simulink model "pfb_fft_vacc_float"  (R2016b)
settings: Solver=VariableStepAuto  StartTime=0.0  StopTime=1024*2^5  RelTol=1e-3
undefined symbols (not defined in the file): fft_stages
libraries: xbsIndex_r4 x15685, casper_library_misc x36, casper_library_delays x15, casper_library_sources x4, xbsReplacements_r4 x3, xps_library x1
(part 38 of 134)

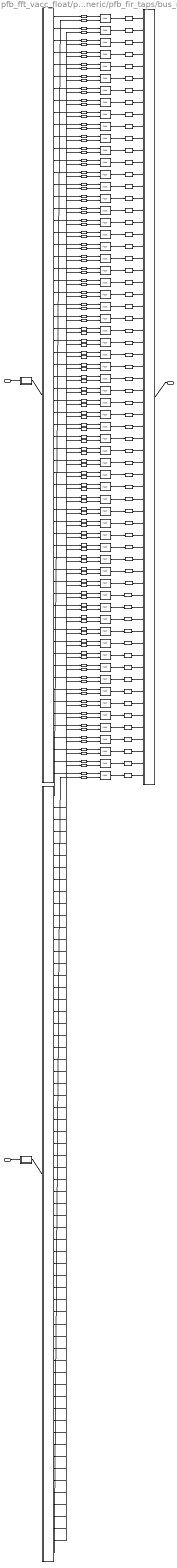
== system pfb_fft_vacc_float/pfb/pfb_fir_generic/pfb_fir_taps/bus_mult ==
  a : Inport
  b : Inport  Port=2
  Convert{,1..127} (128 alike) : xbsIndex_r4/Convert  gui_display_data_type=Floating-point  arith_type=Floating-point  n_bits=16  bin_pt=14  float_type=Single  exp_bits=8  fraction_bits=24  quantization=Truncate  overflow=Wrap  en=off  latency=4  pipeline=on  dbl_ovrd=off  block_type=convert
  Reinterpret{,1..63} (64 alike) : xbsIndex_r4/Reinterpret  force_arith_type=on  arith_type=Unsigned  force_bin_pt=on  bin_pt=0  block_type=reinterpret
  a*b_bussify : Subsystem  AncestorBlock=casper_library_flow_control/bus_create  mask(bus_create){inputNum=64}
      mask prompts: inputNum "Number of inputs:"
      mask init:
      | bus_create_init(gcb,...
      |     'inputNum', inputNum);
  a_debus : Subsystem  AncestorBlock=casper_library_flow_control/bus_expand  mask(bus_expand){mode=divisions of arbitrary size, outputNum=1, outputWidth=[10 10 10 10 10 10 10 10 10 10 10 10 10 10 10 10 10 10 10 10 10 10 10 10 10 10 10 10 10 10 10 10 10 10 10 10 10 10 10 10 10 10 10 10 10 10 10 10 10 10 10 10 10 10 10 10 10 10 10 10 10 10 10 10], outputBinaryPt=[9 9 9 9 9 9 9 9 9 9 9 9 9 9 9 9 9 9 9 9 9 9 9 9 9 9 9 9 9 9 9 9 9 9 9 9 9 9 9 9 9 9 9 9 9 9 9 9 9 9 9 9 9 9 9 9 9 9 9 9 9 9 9 9], outputArithmeticType=[1 1 1 1 1 1 1 1 1 1 1 1 1 1 1 1 1 1 1 1 1 1 1 1 1 1 1 1 1 1 1 1 1 1 1 1 1 1 1 1 1 1 1 1 1 1 1 1 1 1 1 1 1 1 1 1 1 1 1 1 1 1 1 1], show_format=on, outputToWorkspace=off, variablePrefix=, outputToModelAsWell=on}
      mask prompts: outputNum "Number of outputs:"; outputWidth "Output width [msb ... lsb]:"; outputBinaryPt "Output binary point position [msb ... lsb]:"; outputArithmeticType "Output arithmetic type (ufix=0, fix=1, bool=2) [msb ... lsb]:"; show_format "Print format string?"; outputToWorkspace "Output to workspace?"; variablePrefix "Variable name prefix:"; outputToModelAsWell "Output to model as well?"
      mask help:
      | Expands a 'bus' made using a bus_create or Xilinx
      | concat block.
      | The input will be divided into the specified number
      | of outputs with the specified arithmetic types.
      | Divisions are made from most significant bit.
      mask init: same as pfb_fft_vacc_float/bus_expand1
      settings: AttributesFormatString=64 outputs: f10.9,f10.9,f10.9,f10.9,f10.9,f10.9,f10.9,f10.9,f10.9,f10.9,f10.9,f10.9,f10.9,f10.9,f10.9,f10.9,f10.9,f10.9,f10.9,f10.9,f10.9,f10.9,f10.9,f10.9,f10.9,f10.9,f10.9,f10.9,f10.9,f10.9,f10.9,f10.9,f10.9,f10.9,f10.9,f10.9,f10.9,f10.9,f10.9,f10.9,f10.9,f10.9,f10.9,f10.9,f10.9,f10.9,f10.9,f10.9,f10.9,f10.9,f10.9,f10.9,f10.9,f10.9,f10.9,f10.9,f10.9,f10.9,f10.9,f10.9,f10.9,f10.9,f10.9,f10.9,
      mask TypeOptions mode: divisions of equal size | divisions of arbitrary size
      mask Callback mode: bus_expand_callback();
      mask Callback outputWidth: bus_expand_callback();
      mask Callback outputToWorkspace: bus_expand_callback();
  b_debus : Subsystem  AncestorBlock=casper_library_flow_control/bus_expand  mask(bus_expand){mode=divisions of arbitrary size, outputNum=1, outputWidth=[18 18 18 18 18 18 18 18 18 18 18 18 18 18 18 18 18 18 18 18 18 18 18 18 18 18 18 18 18 18 18 18 18 18 18 18 18 18 18 18 18 18 18 18 18 18 18 18 18 18 18 18 18 18 18 18 18 18 18 18 18 18 18 18], outputBinaryPt=[17 17 17 17 17 17 17 17 17 17 17 17 17 17 17 17 17 17 17 17 17 17 17 17 17 17 17 17 17 17 17 17 17 17 17 17 17 17 17 17 17 17 17 17 17 17 17 17 17 17 17 17 17 17 17 17 17 17 17 17 17 17 17 17], outputArithmeticType=[1 1 1 1 1 1 1 1 1 1 1 1 1 1 1 1 1 1 1 1 1 1 1 1 1 1 1 1 1 1 1 1 1 1 1 1 1 1 1 1 1 1 1 1 1 1 1 1 1 1 1 1 1 1 1 1 1 1 1 1 1 1 1 1], show_format=on, outputToWorkspace=off, variablePrefix=, outputToModelAsWell=on}
      mask prompts: outputNum "Number of outputs:"; outputWidth "Output width [msb ... lsb]:"; outputBinaryPt "Output binary point position [msb ... lsb]:"; outputArithmeticType "Output arithmetic type (ufix=0, fix=1, bool=2) [msb ... lsb]:"; show_format "Print format string?"; outputToWorkspace "Output to workspace?"; variablePrefix "Variable name prefix:"; outputToModelAsWell "Output to model as well?"
      mask help:
      | Expands a 'bus' made using a bus_create or Xilinx
      | concat block.
      | The input will be divided into the specified number
      | of outputs with the specified arithmetic types.
      | Divisions are made from most significant bit.
      mask init: same as pfb_fft_vacc_float/bus_expand1
      settings: AttributesFormatString=64 outputs: f18.17,f18.17,f18.17,f18.17,f18.17,f18.17,f18.17,f18.17,f18.17,f18.17,f18.17,f18.17,f18.17,f18.17,f18.17,f18.17,f18.17,f18.17,f18.17,f18.17,f18.17,f18.17,f18.17,f18.17,f18.17,f18.17,f18.17,f18.17,f18.17,f18.17,f18.17,f18.17,f18.17,f18.17,f18.17,f18.17,f18.17,f18.17,f18.17,f18.17,f18.17,f18.17,f18.17,f18.17,f18.17,f18.17,f18.17,f18.17,f18.17,f18.17,f18.17,f18.17,f18.17,f18.17,f18.17,f18.17,f18.17,f18.17,f18.17,f18.17,f18.17,f18.17,f18.17,f18.17,
      mask TypeOptions mode: divisions of equal size | divisions of arbitrary size
      mask Callback mode: bus_expand_callback();
      mask Callback outputWidth: bus_expand_callback();
      mask Callback outputToWorkspace: bus_expand_callback();
  mult{1..64} (64 alike) : xbsIndex_r4/Mult  precision=Full  arith_type="Signed  (2's comp)"  n_bits=28  bin_pt=26  quantization=Truncate  overflow=Wrap  en=off  latency=mult_latency+3  use_behavioral_HDL=off  opt=Speed  use_embedded=on  optimum_pipeline=off  pipeline=on  dbl_ovrd=off  use_rpm=on  placement_style=Triangular  block_type=mult
  repa : Subsystem  AncestorBlock=casper_library_bus/bus_replicate  mask(bus_replicate){replication=1, latency=0, misc=off, implementation=core}
      mask prompts: replication "replication factor"; misc "misc support"; implementation "delay implementation"
      mask help: Output bus formed by replicating input bus a number of times
      mask init:
      | bus_replicate_init(gcb, ...
      |     'replication', replication, ...
      |     'latency', latency, ...
      |     'implementation', implementation, ...
      |     'misc', misc);
      mask TypeOptions implementation: core | behavioral
  repb : Subsystem  AncestorBlock=casper_library_bus/bus_replicate  mask(bus_replicate){replication=1, latency=0, misc=off, implementation=core}
      mask prompts: replication "replication factor"; misc "misc support"; implementation "delay implementation"
      mask help: Output bus formed by replicating input bus a number of times
      mask init:
      | bus_replicate_init(gcb, ...
      |     'replication', replication, ...
      |     'latency', latency, ...
      |     'implementation', implementation, ...
      |     'misc', misc);
      mask TypeOptions implementation: core | behavioral
  a*b : Outport
  wires:
  a/1 -> repa/1(in)
  b/1 -> repb/1(in)
  repa/1(out) -> a_debus/1(bus_in)
  repb/1(out) -> b_debus/1(bus_in)
  a_debus/1(msb_out64) -> Convert/1
  b_debus/1(msb_out64) -> Convert1/1
  a_debus/2(out63) -> Convert2/1
  b_debus/2(out63) -> Convert3/1
  a_debus/3(out62) -> Convert4/1
  b_debus/3(out62) -> Convert5/1
  a_debus/4(out61) -> Convert6/1
  b_debus/4(out61) -> Convert7/1
  a_debus/5(out60) -> Convert8/1
  b_debus/5(out60) -> Convert9/1
  a_debus/6(out59) -> Convert10/1
  b_debus/6(out59) -> Convert11/1
  a_debus/7(out58) -> Convert12/1
  b_debus/7(out58) -> Convert13/1
  a_debus/8(out57) -> Convert14/1
  b_debus/8(out57) -> Convert15/1
  a_debus/9(out56) -> Convert16/1
  b_debus/9(out56) -> Convert17/1
  a_debus/10(out55) -> Convert24/1
  b_debus/10(out55) -> Convert25/1
  a_debus/11(out54) -> Convert26/1
  b_debus/11(out54) -> Convert27/1
  a_debus/12(out53) -> Convert28/1
  b_debus/12(out53) -> Convert29/1
  a_debus/13(out52) -> Convert30/1
  b_debus/13(out52) -> Convert31/1
  a_debus/14(out51) -> Convert18/1
  b_debus/14(out51) -> Convert19/1
  a_debus/15(out50) -> Convert20/1
  b_debus/15(out50) -> Convert21/1
  a_debus/16(out49) -> Convert22/1
  b_debus/16(out49) -> Convert23/1
  a_debus/17(out48) -> Convert32/1
  b_debus/17(out48) -> Convert33/1
  a_debus/18(out47) -> Convert40/1
  b_debus/18(out47) -> Convert41/1
  a_debus/19(out46) -> Convert42/1
  b_debus/19(out46) -> Convert43/1
  a_debus/20(out45) -> Convert44/1
  b_debus/20(out45) -> Convert45/1
  a_debus/21(out44) -> Convert46/1
  b_debus/21(out44) -> Convert47/1
  a_debus/22(out43) -> Convert34/1
  b_debus/22(out43) -> Convert35/1
  a_debus/23(out42) -> Convert36/1
  b_debus/23(out42) -> Convert37/1
  a_debus/24(out41) -> Convert38/1
  b_debus/24(out41) -> Convert39/1
  a_debus/25(out40) -> Convert48/1
  b_debus/25(out40) -> Convert49/1
  a_debus/26(out39) -> Convert56/1
  b_debus/26(out39) -> Convert57/1
  a_debus/27(out38) -> Convert58/1
  b_debus/27(out38) -> Convert59/1
  a_debus/28(out37) -> Convert60/1
  b_debus/28(out37) -> Convert61/1
  a_debus/29(out36) -> Convert62/1
  b_debus/29(out36) -> Convert63/1
  a_debus/30(out35) -> Convert50/1
  b_debus/30(out35) -> Convert51/1
  a_debus/31(out34) -> Convert52/1
  b_debus/31(out34) -> Convert53/1
  a_debus/32(out33) -> Convert54/1
  b_debus/32(out33) -> Convert55/1
  a_debus/33(out32) -> Convert64/1
  b_debus/33(out32) -> Convert65/1
  a_debus/34(out31) -> Convert72/1
  b_debus/34(out31) -> Convert73/1
  a_debus/35(out30) -> Convert74/1
  b_debus/35(out30) -> Convert75/1
  a_debus/36(out29) -> Convert76/1
  b_debus/36(out29) -> Convert77/1
  a_debus/37(out28) -> Convert78/1
  b_debus/37(out28) -> Convert79/1
  a_debus/38(out27) -> Convert66/1
  b_debus/38(out27) -> Convert67/1
  a_debus/39(out26) -> Convert68/1
  b_debus/39(out26) -> Convert69/1
  a_debus/40(out25) -> Convert70/1
  b_debus/40(out25) -> Convert71/1
  a_debus/41(out24) -> Convert80/1
  b_debus/41(out24) -> Convert81/1
  a_debus/42(out23) -> Convert88/1
  b_debus/42(out23) -> Convert89/1
  a_debus/43(out22) -> Convert90/1
  b_debus/43(out22) -> Convert91/1
  a_debus/44(out21) -> Convert92/1
  b_debus/44(out21) -> Convert93/1
  a_debus/45(out20) -> Convert94/1
  b_debus/45(out20) -> Convert95/1
  a_debus/46(out19) -> Convert82/1
  b_debus/46(out19) -> Convert83/1
  a_debus/47(out18) -> Convert84/1
  b_debus/47(out18) -> Convert85/1
  a_debus/48(out17) -> Convert86/1
  b_debus/48(out17) -> Convert87/1
  a_debus/49(out16) -> Convert96/1
  b_debus/49(out16) -> Convert97/1
  a_debus/50(out15) -> Convert104/1
  b_debus/50(out15) -> Convert105/1
  a_debus/51(out14) -> Convert106/1
  b_debus/51(out14) -> Convert107/1
  a_debus/52(out13) -> Convert108/1
  b_debus/52(out13) -> Convert109/1
  a_debus/53(out12) -> Convert110/1
  b_debus/53(out12) -> Convert111/1
  a_debus/54(out11) -> Convert98/1
  b_debus/54(out11) -> Convert99/1
  a_debus/55(out10) -> Convert100/1
  b_debus/55(out10) -> Convert101/1
  a_debus/56(out9) -> Convert102/1
  b_debus/56(out9) -> Convert103/1
  a_debus/57(out8) -> Convert124/1
  b_debus/57(out8) -> Convert125/1
  a_debus/58(out7) -> Convert116/1
  b_debus/58(out7) -> Convert117/1
  a_debus/59(out6) -> Convert118/1
  b_debus/59(out6) -> Convert119/1
  a_debus/60(out5) -> Convert120/1
  b_debus/60(out5) -> Convert121/1
  a_debus/61(out4) -> Convert122/1
  b_debus/61(out4) -> Convert123/1
  a_debus/62(out3) -> Convert126/1
  b_debus/62(out3) -> Convert127/1
  a_debus/63(out2) -> Convert112/1
  b_debus/63(out2) -> Convert113/1
  a_debus/64(lsb_out1) -> Convert114/1
  b_debus/64(lsb_out1) -> Convert115/1
  mult2/1 -> Reinterpret1/1
  mult3/1 -> Reinterpret2/1
  mult4/1 -> Reinterpret3/1
  mult5/1 -> Reinterpret4/1
  mult6/1 -> Reinterpret5/1
  mult7/1 -> Reinterpret6/1
  mult8/1 -> Reinterpret7/1
  mult9/1 -> Reinterpret8/1
  mult10/1 -> Reinterpret9/1
  mult11/1 -> Reinterpret10/1
  mult12/1 -> Reinterpret11/1
  mult13/1 -> Reinterpret12/1
  mult14/1 -> Reinterpret13/1
  mult15/1 -> Reinterpret14/1
  mult16/1 -> Reinterpret15/1
  mult24/1 -> Reinterpret22/1
  mult17/1 -> Reinterpret23/1
  mult18/1 -> Reinterpret16/1
  mult19/1 -> Reinterpret17/1
  mult20/1 -> Reinterpret18/1
  mult21/1 -> Reinterpret19/1
  mult22/1 -> Reinterpret20/1
  mult23/1 -> Reinterpret21/1
  mult32/1 -> Reinterpret30/1
  mult25/1 -> Reinterpret31/1
  mult26/1 -> Reinterpret24/1
  mult27/1 -> Reinterpret25/1
  mult28/1 -> Reinterpret26/1
  mult29/1 -> Reinterpret27/1
  mult30/1 -> Reinterpret28/1
  mult31/1 -> Reinterpret29/1
  mult40/1 -> Reinterpret38/1
  mult33/1 -> Reinterpret39/1
  mult34/1 -> Reinterpret32/1
  mult35/1 -> Reinterpret33/1
  mult36/1 -> Reinterpret34/1
  mult37/1 -> Reinterpret35/1
  mult38/1 -> Reinterpret36/1
  mult39/1 -> Reinterpret37/1
  mult48/1 -> Reinterpret46/1
  mult41/1 -> Reinterpret47/1
  mult42/1 -> Reinterpret40/1
  mult43/1 -> Reinterpret41/1
  mult44/1 -> Reinterpret42/1
  mult45/1 -> Reinterpret43/1
  mult46/1 -> Reinterpret44/1
  mult47/1 -> Reinterpret45/1
  mult56/1 -> Reinterpret54/1
  mult49/1 -> Reinterpret55/1
  mult50/1 -> Reinterpret48/1
  mult51/1 -> Reinterpret49/1
  mult52/1 -> Reinterpret50/1
  mult53/1 -> Reinterpret51/1
  mult54/1 -> Reinterpret52/1
  mult55/1 -> Reinterpret53/1
  mult57/1 -> Reinterpret62/1
  mult58/1 -> Reinterpret63/1
  mult59/1 -> Reinterpret56/1
  mult60/1 -> Reinterpret57/1
  mult61/1 -> Reinterpret58/1
  mult62/1 -> Reinterpret59/1
  mult63/1 -> Reinterpret60/1
  mult64/1 -> Reinterpret61/1
  a*b_bussify/1(bus_out) -> a*b/1
  Convert/1 -> mult1/1
  Convert1/1 -> mult1/2
  Convert3/1 -> mult2/2
  Convert2/1 -> mult2/1
  Convert5/1 -> mult3/2
  Convert4/1 -> mult3/1
  Convert7/1 -> mult4/2
  Convert6/1 -> mult4/1
  Convert14/1 -> mult8/1
  Convert11/1 -> mult6/2
  Convert10/1 -> mult6/1
  Convert13/1 -> mult7/2
  Convert12/1 -> mult7/1
  Convert8/1 -> mult5/1
  Convert15/1 -> mult8/2
  Convert9/1 -> mult5/2
  Convert31/1 -> mult13/2
  Convert19/1 -> mult14/2
  Convert16/1 -> mult9/1
  Convert17/1 -> mult9/2
  Convert26/1 -> mult11/1
  Convert29/1 -> mult12/2
  Convert18/1 -> mult14/1
  Convert23/1 -> mult16/2
  Convert22/1 -> mult16/1
  Convert27/1 -> mult11/2
  Convert21/1 -> mult15/2
  Convert24/1 -> mult10/1
  Convert20/1 -> mult15/1
  Convert28/1 -> mult12/1
  Convert25/1 -> mult10/2
  Convert30/1 -> mult13/1
  Convert33/1 -> mult24/2
  Convert45/1 -> mult19/2
  Convert38/1 -> mult23/1
  Convert41/1 -> mult17/2
  Convert34/1 -> mult21/1
  Convert46/1 -> mult20/1
  Convert32/1 -> mult24/1
  Convert43/1 -> mult18/2
  Convert39/1 -> mult23/2
  Convert37/1 -> mult22/2
  Convert42/1 -> mult18/1
  Convert40/1 -> mult17/1
  Convert36/1 -> mult22/1
  Convert44/1 -> mult19/1
  Convert47/1 -> mult20/2
  Convert35/1 -> mult21/2
  Convert55/1 -> mult31/2
  Convert61/1 -> mult27/2
  Convert58/1 -> mult26/1
  Convert52/1 -> mult30/1
  Convert48/1 -> mult32/1
  Convert51/1 -> mult29/2
  Convert53/1 -> mult30/2
  Convert62/1 -> mult28/1
  Convert49/1 -> mult32/2
  Convert60/1 -> mult27/1
  Convert56/1 -> mult25/1
  Convert57/1 -> mult25/2
  Convert54/1 -> mult31/1
  Convert59/1 -> mult26/2
  Convert50/1 -> mult29/1
  Convert63/1 -> mult28/2
  Convert79/1 -> mult36/2
  Convert74/1 -> mult34/1
  Convert78/1 -> mult36/1
  Convert77/1 -> mult35/2
  Convert65/1 -> mult40/2
  Convert71/1 -> mult39/2
  Convert64/1 -> mult40/1
  Convert69/1 -> mult38/2
  Convert70/1 -> mult39/1
  Convert76/1 -> mult35/1
  Convert66/1 -> mult37/1
  Convert67/1 -> mult37/2
  Convert68/1 -> mult38/1
  Convert73/1 -> mult33/2
  Convert75/1 -> mult34/2
  Convert72/1 -> mult33/1
  Convert80/1 -> mult48/1
  Convert91/1 -> mult42/2
  Convert89/1 -> mult41/2
  Convert87/1 -> mult47/2
  Convert94/1 -> mult44/1
  Convert88/1 -> mult41/1
  Convert93/1 -> mult43/2
  Convert95/1 -> mult44/2
  Convert81/1 -> mult48/2
  Convert92/1 -> mult43/1
  Convert86/1 -> mult47/1
  Convert84/1 -> mult46/1
  Convert85/1 -> mult46/2
  Convert90/1 -> mult42/1
  Convert83/1 -> mult45/2
  Convert82/1 -> mult45/1
  Convert108/1 -> mult51/1
  Convert106/1 -> mult50/1
  Convert98/1 -> mult53/1
  Convert102/1 -> mult55/1
  Convert104/1 -> mult49/1
  Convert105/1 -> mult49/2
  Convert110/1 -> mult52/1
  Convert109/1 -> mult51/2
  Convert111/1 -> mult52/2
  Convert100/1 -> mult54/1
  Convert96/1 -> mult56/1
  Convert97/1 -> mult56/2
  Convert107/1 -> mult50/2
  Convert101/1 -> mult54/2
  Convert103/1 -> mult55/2
  Convert99/1 -> mult53/2
  Convert126/1 -> mult62/1
  Convert127/1 -> mult62/2
  Convert115/1 -> mult64/2
  Convert118/1 -> mult59/1
  Convert116/1 -> mult58/1
  Convert117/1 -> mult58/2
  Convert125/1 -> mult57/2
  Convert114/1 -> mult64/1
  Convert119/1 -> mult59/2
  Convert122/1 -> mult61/1
  Convert112/1 -> mult63/1
  Convert121/1 -> mult60/2
  Convert124/1 -> mult57/1
  Convert123/1 -> mult61/2
  Convert120/1 -> mult60/1
  Convert113/1 -> mult63/2
  Reinterpret/1 -> a*b_bussify/1(in1)
  Reinterpret1/1 -> a*b_bussify/2(in2)
  Reinterpret2/1 -> a*b_bussify/3(in3)
  Reinterpret3/1 -> a*b_bussify/4(in4)
  Reinterpret4/1 -> a*b_bussify/5(in5)
  Reinterpret7/1 -> a*b_bussify/8(in8)
  Reinterpret6/1 -> a*b_bussify/7(in7)
  Reinterpret5/1 -> a*b_bussify/6(in6)
  Reinterpret13/1 -> a*b_bussify/14(in14)
  Reinterpret15/1 -> a*b_bussify/16(in16)
  Reinterpret11/1 -> a*b_bussify/12(in12)
  Reinterpret9/1 -> a*b_bussify/10(in10)
  Reinterpret14/1 -> a*b_bussify/15(in15)
  Reinterpret8/1 -> a*b_bussify/9(in9)
  Reinterpret12/1 -> a*b_bussify/13(in13)
  Reinterpret10/1 -> a*b_bussify/11(in11)
  Reinterpret16/1 -> a*b_bussify/19(in19)
  Reinterpret21/1 -> a*b_bussify/24(in24)
  Reinterpret17/1 -> a*b_bussify/20(in20)
  Reinterpret18/1 -> a*b_bussify/21(in21)
  Reinterpret22/1 -> a*b_bussify/17(in17)
  Reinterpret23/1 -> a*b_bussify/18(in18)
  Reinterpret20/1 -> a*b_bussify/23(in23)
  Reinterpret19/1 -> a*b_bussify/22(in22)
  Reinterpret24/1 -> a*b_bussify/27(in27)
  Reinterpret30/1 -> a*b_bussify/25(in25)
  Reinterpret26/1 -> a*b_bussify/29(in29)
  Reinterpret27/1 -> a*b_bussify/30(in30)
  Reinterpret28/1 -> a*b_bussify/31(in31)
  Reinterpret31/1 -> a*b_bussify/26(in26)
  Reinterpret29/1 -> a*b_bussify/32(in32)
  Reinterpret25/1 -> a*b_bussify/28(in28)
  Reinterpret34/1 -> a*b_bussify/37(in37)
  Reinterpret36/1 -> a*b_bussify/39(in39)
  Reinterpret35/1 -> a*b_bussify/38(in38)
  Reinterpret38/1 -> a*b_bussify/33(in33)
  Reinterpret32/1 -> a*b_bussify/35(in35)
  Reinterpret33/1 -> a*b_bussify/36(in36)
  Reinterpret37/1 -> a*b_bussify/40(in40)
  Reinterpret39/1 -> a*b_bussify/34(in34)
  Reinterpret41/1 -> a*b_bussify/44(in44)
  Reinterpret44/1 -> a*b_bussify/47(in47)
  Reinterpret42/1 -> a*b_bussify/45(in45)
  Reinterpret46/1 -> a*b_bussify/41(in41)
  Reinterpret40/1 -> a*b_bussify/43(in43)
  Reinterpret47/1 -> a*b_bussify/42(in42)
  Reinterpret45/1 -> a*b_bussify/48(in48)
  Reinterpret43/1 -> a*b_bussify/46(in46)
  Reinterpret51/1 -> a*b_bussify/54(in54)
  Reinterpret54/1 -> a*b_bussify/49(in49)
  Reinterpret55/1 -> a*b_bussify/50(in50)
  Reinterpret48/1 -> a*b_bussify/51(in51)
  Reinterpret53/1 -> a*b_bussify/56(in56)
  Reinterpret50/1 -> a*b_bussify/53(in53)
  Reinterpret52/1 -> a*b_bussify/55(in55)
  Reinterpret49/1 -> a*b_bussify/52(in52)
  Reinterpret63/1 -> a*b_bussify/58(in58)
  Reinterpret56/1 -> a*b_bussify/59(in59)
  Reinterpret61/1 -> a*b_bussify/64(in64)
  Reinterpret60/1 -> a*b_bussify/63(in63)
  Reinterpret57/1 -> a*b_bussify/60(in60)
  Reinterpret59/1 -> a*b_bussify/62(in62)
  Reinterpret62/1 -> a*b_bussify/57(in57)
  Reinterpret58/1 -> a*b_bussify/61(in61)
  mult1/1 -> Reinterpret/1

== system pfb_fft_vacc_float/pfb/pfb_fir_generic/pfb_fir_taps/pipeline1 ==
  d : Inport
  Register0 : xbsIndex_r4/Register  init=0  rst=off  en=off  dbl_ovrd=off  block_type=register
      settings: block_version=11.4
  Register1 : xbsIndex_r4/Register  init=0  rst=off  en=off  dbl_ovrd=off  block_type=register
  q : Outport
  wires:
  Register1/1 -> q/1
  d/1 -> Register0/1
  Register0/1 -> Register1/1

== system pfb_fft_vacc_float/pfb/pfb_fir_generic/pfb_fir_taps/pipeline2 ==
  d : Inport
  Register0 : xbsIndex_r4/Register  init=0  rst=off  en=off  dbl_ovrd=off  block_type=register
      settings: block_version=11.4
  Register1 : xbsIndex_r4/Register  init=0  rst=off  en=off  dbl_ovrd=off  block_type=register
  q : Outport
  wires:
  Register0/1 -> Register1/1
  d/1 -> Register0/1
  Register1/1 -> q/1

== system pfb_fft_vacc_float/pfb/pfb_fir_generic/pfb_fir_taps/pipeline3 ==
  d : Inport
  Register0 : xbsIndex_r4/Register  init=0  rst=off  en=off  dbl_ovrd=off  block_type=register
      settings: block_version=11.4
  Register1 : xbsIndex_r4/Register  init=0  rst=off  en=off  dbl_ovrd=off  block_type=register
  q : Outport
  wires:
  Register1/1 -> q/1
  d/1 -> Register0/1
  Register0/1 -> Register1/1

== system pfb_fft_vacc_float/pfb/pfb_fir_generic/pfb_fir_taps/pipeline8 ==
  d : Inport
  Register0 : xbsIndex_r4/Register  init=0  rst=off  en=off  dbl_ovrd=off  block_type=register
      settings: block_version=11.4
  Register1 : xbsIndex_r4/Register  init=0  rst=off  en=off  dbl_ovrd=off  block_type=register
  q : Outport
  wires:
  Register0/1 -> Register1/1
  d/1 -> Register0/1
  Register1/1 -> q/1

== system pfb_fft_vacc_float/pfb/pfb_fir_generic/pfb_fir_taps/tap_delays ==
  addra : Inport
  dina : Inport  Port=2
  wea : Inport  Port=3
  addrb : Inport  Port=4
  dinb : Inport  Port=5
  web : Inport  Port=6
  A_bussify0 : Subsystem  AncestorBlock=casper_library_flow_control/bus_create  mask(bus_create){inputNum=14}
      mask prompts: inputNum "Number of inputs:"
      mask init:
      | bus_create_init(gcb,...
      |     'inputNum', inputNum);
  B_bussify0 : Subsystem  AncestorBlock=casper_library_flow_control/bus_create  mask(bus_create){inputNum=14}
      mask prompts: inputNum "Number of inputs:"
      mask init:
      | bus_create_init(gcb,...
      |     'inputNum', inputNum);
  alsbs_slice : xbsIndex_r4/Slice  nbits=10  boolean_output=off  mode=Lower Bit Location + Width  bit1=0  base1=LSB of Input  bit0=0  base0=LSB of Input  dbl_ovrd=off  block_type=slice
  blsbs_slice : xbsIndex_r4/Slice  nbits=10  boolean_output=off  mode=Lower Bit Location + Width  bit1=0  base1=LSB of Input  bit0=0  base0=LSB of Input  dbl_ovrd=off  block_type=slice
  bram0_{0..13} (14 alike) : xbsIndex_r4/Dual Port RAM  depth=1024  initVector=getfield(get_param(gcb, 'UserData'), 'initVector')  distributed_mem=Block RAM  init_a=0  init_b=0  rst_a=off  rst_b=off  en_a=off  en_b=off  latency=4  write_mode_A=Read Before Write  write_mode_B=Read Before Write  optimize=Speed  dbl_ovrd=off  block_type=dpram
  ddina0 : xbsIndex_r4/Delay  rst=off  infoeditControl=Selection of Reset will increase slice count due to use of real FFs and instead of SRLs  en=off  latency=0  reg_retiming=on  dbl_ovrd=off  block_type=delay
      settings: infoedit=Hardware notes: A delay line is a chain, each link of which is an SRL16 or SRL32 followed by a flip-flop.
  ddinb0 : xbsIndex_r4/Delay  rst=off  infoeditControl=Selection of Reset will increase slice count due to use of real FFs and instead of SRLs  en=off  latency=6  reg_retiming=off  dbl_ovrd=off  block_type=delay
      settings: infoedit=Hardware notes: A delay line is a chain, each link of which is an SRL16 or SRL32 followed by a flip-flop.
  debus_addra0 : Subsystem  AncestorBlock=casper_library_flow_control/bus_expand  mask(bus_expand){mode=divisions of equal size, outputNum=14, outputWidth=[10 10 10 10 10 10 10 10 10 10 10 10 10 10], outputBinaryPt=[0 0 0 0 0 0 0 0 0 0 0 0 0 0], outputArithmeticType=[0 0 0 0 0 0 0 0 0 0 0 0 0 0], show_format=on, outputToWorkspace=off, variablePrefix=, outputToModelAsWell=on}
      mask prompts: outputNum "Number of outputs:"; outputWidth "Output width:"; outputBinaryPt "Output binary point position:"; outputArithmeticType "Output arithmetic type (ufix=0, fix=1, bool=2):"; show_format "Print format string?"; outputToWorkspace "Output to workspace?"; variablePrefix "Variable name prefix:"; outputToModelAsWell "Output to model as well?"
      mask help:
      | Expands a 'bus' made using a bus_create or Xilinx
      | concat block.
      | The input will be divided into the specified number
      | of outputs with the specified arithmetic types.
      | Divisions are made from most significant bit.
      mask init: same as pfb_fft_vacc_float/bus_expand1
      settings: AttributesFormatString=14 outputs: uf10.0,uf10.0,uf10.0,uf10.0,uf10.0,uf10.0,uf10.0,uf10.0,uf10.0,uf10.0,uf10.0,uf10.0,uf10.0,uf10.0,
      mask TypeOptions mode: divisions of equal size | divisions of arbitrary size
      mask Callback mode: bus_expand_callback();
      mask Callback outputWidth: bus_expand_callback();
      mask Callback outputToWorkspace: bus_expand_callback();
  debus_addrb0 : Subsystem  AncestorBlock=casper_library_flow_control/bus_expand  mask(bus_expand){mode=divisions of equal size, outputNum=14, outputWidth=[10 10 10 10 10 10 10 10 10 10 10 10 10 10], outputBinaryPt=[0 0 0 0 0 0 0 0 0 0 0 0 0 0], outputArithmeticType=[0 0 0 0 0 0 0 0 0 0 0 0 0 0], show_format=on, outputToWorkspace=off, variablePrefix=, outputToModelAsWell=on}
      mask prompts: outputNum "Number of outputs:"; outputWidth "Output width:"; outputBinaryPt "Output binary point position:"; outputArithmeticType "Output arithmetic type (ufix=0, fix=1, bool=2):"; show_format "Print format string?"; outputToWorkspace "Output to workspace?"; variablePrefix "Variable name prefix:"; outputToModelAsWell "Output to model as well?"
      mask help:
      | Expands a 'bus' made using a bus_create or Xilinx
      | concat block.
      | The input will be divided into the specified number
      | of outputs with the specified arithmetic types.
      | Divisions are made from most significant bit.
      mask init: same as pfb_fft_vacc_float/bus_expand1
      settings: AttributesFormatString=14 outputs: uf10.0,uf10.0,uf10.0,uf10.0,uf10.0,uf10.0,uf10.0,uf10.0,uf10.0,uf10.0,uf10.0,uf10.0,uf10.0,uf10.0,
      mask TypeOptions mode: divisions of equal size | divisions of arbitrary size
      mask Callback mode: bus_expand_callback();
      mask Callback outputWidth: bus_expand_callback();
      mask Callback outputToWorkspace: bus_expand_callback();
  debus_dina0 : Subsystem  AncestorBlock=casper_library_flow_control/bus_expand  mask(bus_expand){mode=divisions of arbitrary size, outputNum=1, outputWidth=[12 36 36 36 36 36 36 36 36 36 36 36 36 36], outputBinaryPt=[0 0 0 0 0 0 0 0 0 0 0 0 0 0], outputArithmeticType=[0 0 0 0 0 0 0 0 0 0 0 0 0 0], show_format=on, outputToWorkspace=off, variablePrefix=, outputToModelAsWell=on}
      mask prompts: outputNum "Number of outputs:"; outputWidth "Output width [msb ... lsb]:"; outputBinaryPt "Output binary point position [msb ... lsb]:"; outputArithmeticType "Output arithmetic type (ufix=0, fix=1, bool=2) [msb ... lsb]:"; show_format "Print format string?"; outputToWorkspace "Output to workspace?"; variablePrefix "Variable name prefix:"; outputToModelAsWell "Output to model as well?"
      mask help:
      | Expands a 'bus' made using a bus_create or Xilinx
      | concat block.
      | The input will be divided into the specified number
      | of outputs with the specified arithmetic types.
      | Divisions are made from most significant bit.
      mask init: same as pfb_fft_vacc_float/bus_expand1
      settings: AttributesFormatString=14 outputs: uf12.0,uf36.0,uf36.0,uf36.0,uf36.0,uf36.0,uf36.0,uf36.0,uf36.0,uf36.0,uf36.0,uf36.0,uf36.0,uf36.0,
      mask TypeOptions mode: divisions of equal size | divisions of arbitrary size
      mask Callback mode: bus_expand_callback();
      mask Callback outputWidth: bus_expand_callback();
      mask Callback outputToWorkspace: bus_expand_callback();
  debus_dinb0 : Subsystem  AncestorBlock=casper_library_flow_control/bus_expand  mask(bus_expand){mode=divisions of arbitrary size, outputNum=1, outputWidth=[12 36 36 36 36 36 36 36 36 36 36 36 36 36], outputBinaryPt=[0 0 0 0 0 0 0 0 0 0 0 0 0 0], outputArithmeticType=[0 0 0 0 0 0 0 0 0 0 0 0 0 0], show_format=on, outputToWorkspace=off, variablePrefix=, outputToModelAsWell=on}
      mask prompts: outputNum "Number of outputs:"; outputWidth "Output width [msb ... lsb]:"; outputBinaryPt "Output binary point position [msb ... lsb]:"; outputArithmeticType "Output arithmetic type (ufix=0, fix=1, bool=2) [msb ... lsb]:"; show_format "Print format string?"; outputToWorkspace "Output to workspace?"; variablePrefix "Variable name prefix:"; outputToModelAsWell "Output to model as well?"
      mask help:
      | Expands a 'bus' made using a bus_create or Xilinx
      | concat block.
      | The input will be divided into the specified number
      | of outputs with the specified arithmetic types.
      | Divisions are made from most significant bit.
      mask init: same as pfb_fft_vacc_float/bus_expand1
      settings: AttributesFormatString=14 outputs: uf12.0,uf36.0,uf36.0,uf36.0,uf36.0,uf36.0,uf36.0,uf36.0,uf36.0,uf36.0,uf36.0,uf36.0,uf36.0,uf36.0,
      mask TypeOptions mode: divisions of equal size | divisions of arbitrary size
      mask Callback mode: bus_expand_callback();
      mask Callback outputWidth: bus_expand_callback();
      mask Callback outputToWorkspace: bus_expand_callback();
  debus_wea0 : Subsystem  AncestorBlock=casper_library_flow_control/bus_expand  mask(bus_expand){mode=divisions of equal size, outputNum=14, outputWidth=[1 1 1 1 1 1 1 1 1 1 1 1 1 1], outputBinaryPt=[0 0 0 0 0 0 0 0 0 0 0 0 0 0], outputArithmeticType=[2 2 2 2 2 2 2 2 2 2 2 2 2 2], show_format=on, outputToWorkspace=off, variablePrefix=, outputToModelAsWell=on}
      mask prompts: outputNum "Number of outputs:"; outputWidth "Output width:"; outputBinaryPt "Output binary point position:"; outputArithmeticType "Output arithmetic type (ufix=0, fix=1, bool=2):"; show_format "Print format string?"; outputToWorkspace "Output to workspace?"; variablePrefix "Variable name prefix:"; outputToModelAsWell "Output to model as well?"
      mask help:
      | Expands a 'bus' made using a bus_create or Xilinx
      | concat block.
      | The input will be divided into the specified number
      | of outputs with the specified arithmetic types.
      | Divisions are made from most significant bit.
      mask init: same as pfb_fft_vacc_float/bus_expand1
      settings: AttributesFormatString=14 outputs: b,b,b,b,b,b,b,b,b,b,b,b,b,b,
      mask TypeOptions mode: divisions of equal size | divisions of arbitrary size
      mask Callback mode: bus_expand_callback();
      mask Callback outputWidth: bus_expand_callback();
      mask Callback outputToWorkspace: bus_expand_callback();
  debus_web0 : Subsystem  AncestorBlock=casper_library_flow_control/bus_expand  mask(bus_expand){mode=divisions of equal size, outputNum=14, outputWidth=[1 1 1 1 1 1 1 1 1 1 1 1 1 1], outputBinaryPt=[0 0 0 0 0 0 0 0 0 0 0 0 0 0], outputArithmeticType=[2 2 2 2 2 2 2 2 2 2 2 2 2 2], show_format=on, outputToWorkspace=off, variablePrefix=, outputToModelAsWell=on}
      mask prompts: outputNum "Number of outputs:"; outputWidth "Output width:"; outputBinaryPt "Output binary point position:"; outputArithmeticType "Output arithmetic type (ufix=0, fix=1, bool=2):"; show_format "Print format string?"; outputToWorkspace "Output to workspace?"; variablePrefix "Variable name prefix:"; outputToModelAsWell "Output to model as well?"
      mask help:
      | Expands a 'bus' made using a bus_create or Xilinx
      | concat block.
      | The input will be divided into the specified number
      | of outputs with the specified arithmetic types.
      | Divisions are made from most significant bit.
      mask init: same as pfb_fft_vacc_float/bus_expand1
      settings: AttributesFormatString=14 outputs: b,b,b,b,b,b,b,b,b,b,b,b,b,b,
      mask TypeOptions mode: divisions of equal size | divisions of arbitrary size
      mask Callback mode: bus_expand_callback();
      mask Callback outputWidth: bus_expand_callback();
      mask Callback outputToWorkspace: bus_expand_callback();
  rep_addra0 : Subsystem  AncestorBlock=casper_library_bus/bus_replicate  mask(bus_replicate){replication=14, latency=6, misc=off, implementation=core}
      mask prompts: replication "replication factor"; misc "misc support"; implementation "delay implementation"
      mask help: Output bus formed by replicating input bus a number of times
      mask init:
      | bus_replicate_init(gcb, ...
      |     'replication', replication, ...
      |     'latency', latency, ...
      |     'implementation', implementation, ...
      |     'misc', misc);
      mask TypeOptions implementation: core | behavioral
  rep_addrb0 : Subsystem  AncestorBlock=casper_library_bus/bus_replicate  mask(bus_replicate){replication=14, latency=6, misc=off, implementation=core}
      mask prompts: replication "replication factor"; misc "misc support"; implementation "delay implementation"
      mask help: Output bus formed by replicating input bus a number of times
      mask init:
      | bus_replicate_init(gcb, ...
      |     'replication', replication, ...
      |     'latency', latency, ...
      |     'implementation', implementation, ...
      |     'misc', misc);
      mask TypeOptions implementation: core | behavioral
  rep_wea0 : Subsystem  AncestorBlock=casper_library_bus/bus_replicate  mask(bus_replicate){replication=14, latency=0, misc=off, implementation=behavioral}
      mask prompts: replication "replication factor"; misc "misc support"; implementation "delay implementation"
      mask help: Output bus formed by replicating input bus a number of times
      mask init:
      | bus_replicate_init(gcb, ...
      |     'replication', replication, ...
      |     'latency', latency, ...
      |     'implementation', implementation, ...
      |     'misc', misc);
      mask TypeOptions implementation: core | behavioral
  rep_web0 : Subsystem  AncestorBlock=casper_library_bus/bus_replicate  mask(bus_replicate){replication=14, latency=6, misc=off, implementation=core}
      mask prompts: replication "replication factor"; misc "misc support"; implementation "delay implementation"
      mask help: Output bus formed by replicating input bus a number of times
      mask init:
      | bus_replicate_init(gcb, ...
      |     'replication', replication, ...
      |     'latency', latency, ...
      |     'implementation', implementation, ...
      |     'misc', misc);
      mask TypeOptions implementation: core | behavioral
  A : Outport
  B : Outport  Port=2
  wires:
  addrb/1 -> blsbs_slice/1
  addra/1 -> alsbs_slice/1
  blsbs_slice/1 -> rep_addrb0/1(in)
  alsbs_slice/1 -> rep_addra0/1(in)
  dina/1 -> ddina0/1
  wea/1 -> rep_wea0/1(in)
  dinb/1 -> ddinb0/1
  web/1 -> rep_web0/1(in)
  rep_addrb0/1(out) -> debus_addrb0/1(bus_in)
  rep_addra0/1(out) -> debus_addra0/1(bus_in)
  ddina0/1 -> debus_dina0/1(bus_in)
  rep_wea0/1(out) -> debus_wea0/1(bus_in)
  ddinb0/1 -> debus_dinb0/1(bus_in)
  rep_web0/1(out) -> debus_web0/1(bus_in)
  debus_addra0/1(msb_out14) -> bram0_0/1
  debus_dina0/1(msb_out14) -> bram0_0/2
  debus_wea0/1(msb_out14) -> bram0_0/3
  debus_addrb0/1(msb_out14) -> bram0_0/4
  debus_dinb0/1(msb_out14) -> bram0_0/5
  debus_web0/1(msb_out14) -> bram0_0/6
  debus_addra0/2(out13) -> bram0_1/1
  debus_dina0/2(out13) -> bram0_1/2
  debus_wea0/2(out13) -> bram0_1/3
  debus_addrb0/2(out13) -> bram0_1/4
  debus_dinb0/2(out13) -> bram0_1/5
  debus_web0/2(out13) -> bram0_1/6
  debus_addra0/3(out12) -> bram0_2/1
  debus_dina0/3(out12) -> bram0_2/2
  debus_wea0/3(out12) -> bram0_2/3
  debus_addrb0/3(out12) -> bram0_2/4
  debus_dinb0/3(out12) -> bram0_2/5
  debus_web0/3(out12) -> bram0_2/6
  debus_addra0/4(out11) -> bram0_3/1
  debus_dina0/4(out11) -> bram0_3/2
  debus_wea0/4(out11) -> bram0_3/3
  debus_addrb0/4(out11) -> bram0_3/4
  debus_dinb0/4(out11) -> bram0_3/5
  debus_web0/4(out11) -> bram0_3/6
  debus_addra0/5(out10) -> bram0_4/1
  debus_dina0/5(out10) -> bram0_4/2
  debus_wea0/5(out10) -> bram0_4/3
  debus_addrb0/5(out10) -> bram0_4/4
  debus_dinb0/5(out10) -> bram0_4/5
  debus_web0/5(out10) -> bram0_4/6
  debus_addra0/6(out9) -> bram0_5/1
  debus_dina0/6(out9) -> bram0_5/2
  debus_wea0/6(out9) -> bram0_5/3
  debus_addrb0/6(out9) -> bram0_5/4
  debus_dinb0/6(out9) -> bram0_5/5
  debus_web0/6(out9) -> bram0_5/6
  debus_addra0/7(out8) -> bram0_6/1
  debus_dina0/7(out8) -> bram0_6/2
  debus_wea0/7(out8) -> bram0_6/3
  debus_addrb0/7(out8) -> bram0_6/4
  debus_dinb0/7(out8) -> bram0_6/5
  debus_web0/7(out8) -> bram0_6/6
  debus_addra0/8(out7) -> bram0_7/1
  debus_dina0/8(out7) -> bram0_7/2
  debus_wea0/8(out7) -> bram0_7/3
  debus_addrb0/8(out7) -> bram0_7/4
  debus_dinb0/8(out7) -> bram0_7/5
  debus_web0/8(out7) -> bram0_7/6
  debus_addra0/9(out6) -> bram0_8/1
  debus_dina0/9(out6) -> bram0_8/2
  debus_wea0/9(out6) -> bram0_8/3
  debus_addrb0/9(out6) -> bram0_8/4
  debus_dinb0/9(out6) -> bram0_8/5
  debus_web0/9(out6) -> bram0_8/6
  debus_addra0/10(out5) -> bram0_9/1
  debus_dina0/10(out5) -> bram0_9/2
  debus_wea0/10(out5) -> bram0_9/3
  debus_addrb0/10(out5) -> bram0_9/4
  debus_dinb0/10(out5) -> bram0_9/5
  debus_web0/10(out5) -> bram0_9/6
  debus_addra0/11(out4) -> bram0_10/1
  debus_dina0/11(out4) -> bram0_10/2
  debus_wea0/11(out4) -> bram0_10/3
  debus_addrb0/11(out4) -> bram0_10/4
  debus_dinb0/11(out4) -> bram0_10/5
  debus_web0/11(out4) -> bram0_10/6
  debus_addra0/12(out3) -> bram0_11/1
  debus_dina0/12(out3) -> bram0_11/2
  debus_wea0/12(out3) -> bram0_11/3
  debus_addrb0/12(out3) -> bram0_11/4
  debus_dinb0/12(out3) -> bram0_11/5
  debus_web0/12(out3) -> bram0_11/6
  debus_addra0/13(out2) -> bram0_12/1
  debus_dina0/13(out2) -> bram0_12/2
  debus_wea0/13(out2) -> bram0_12/3
  debus_addrb0/13(out2) -> bram0_12/4
  debus_dinb0/13(out2) -> bram0_12/5
  debus_web0/13(out2) -> bram0_12/6
  debus_addra0/14(lsb_out1) -> bram0_13/1
  debus_dina0/14(lsb_out1) -> bram0_13/2
  debus_wea0/14(lsb_out1) -> bram0_13/3
  debus_addrb0/14(lsb_out1) -> bram0_13/4
  debus_dinb0/14(lsb_out1) -> bram0_13/5
  debus_web0/14(lsb_out1) -> bram0_13/6
  bram0_0/1 -> A_bussify0/1(in1)
  bram0_0/2 -> B_bussify0/1(in1)
  bram0_1/1 -> A_bussify0/2(in2)
  bram0_1/2 -> B_bussify0/2(in2)
  bram0_2/1 -> A_bussify0/3(in3)
  bram0_2/2 -> B_bussify0/3(in3)
  bram0_3/1 -> A_bussify0/4(in4)
  bram0_3/2 -> B_bussify0/4(in4)
  bram0_4/1 -> A_bussify0/5(in5)
  bram0_4/2 -> B_bussify0/5(in5)
  bram0_5/1 -> A_bussify0/6(in6)
  bram0_5/2 -> B_bussify0/6(in6)
  bram0_6/1 -> A_bussify0/7(in7)
  bram0_6/2 -> B_bussify0/7(in7)
  bram0_7/1 -> A_bussify0/8(in8)
  bram0_7/2 -> B_bussify0/8(in8)
  bram0_8/1 -> A_bussify0/9(in9)
  bram0_8/2 -> B_bussify0/9(in9)
  bram0_9/1 -> A_bussify0/10(in10)
  bram0_9/2 -> B_bussify0/10(in10)
  bram0_10/1 -> A_bussify0/11(in11)
  bram0_10/2 -> B_bussify0/11(in11)
  bram0_11/1 -> A_bussify0/12(in12)
  bram0_11/2 -> B_bussify0/12(in12)
  bram0_12/1 -> A_bussify0/13(in13)
  bram0_12/2 -> B_bussify0/13(in13)
  bram0_13/1 -> A_bussify0/14(in14)
  bram0_13/2 -> B_bussify0/14(in14)
  A_bussify0/1(bus_out) -> A/1
  B_bussify0/1(bus_out) -> B/1

== system pfb_fft_vacc_float/pfb/pfb_fir_generic/pfb_fir_taps/tap_split ==
  bus_in : Inport
  reinterpret{1..4} (4 alike) : xbsIndex_r4/Reinterpret  force_arith_type=on  arith_type=Unsigned  force_bin_pt=on  bin_pt=0  block_type=reinterpret
  slice1 : xbsIndex_r4/Slice  nbits=512  boolean_output=off  mode=Upper Bit Location + Width  bit1=-1536  base1=MSB of Input  bit0=0  base0=LSB of Input  dbl_ovrd=off  block_type=slice
  slice2 : xbsIndex_r4/Slice  nbits=512  boolean_output=off  mode=Upper Bit Location + Width  bit1=-1024  base1=MSB of Input  bit0=0  base0=LSB of Input  dbl_ovrd=off  block_type=slice
  slice3 : xbsIndex_r4/Slice  nbits=512  boolean_output=off  mode=Upper Bit Location + Width  bit1=-512  base1=MSB of Input  bit0=0  base0=LSB of Input  dbl_ovrd=off  block_type=slice
  slice4 : xbsIndex_r4/Slice  nbits=512  boolean_output=off  mode=Upper Bit Location + Width  bit1=0  base1=MSB of Input  bit0=0  base0=LSB of Input  dbl_ovrd=off  block_type=slice
  msb_out4 : Outport
  out3 : Outport  Port=2
  out2 : Outport  Port=3
  lsb_out1 : Outport  Port=4
  wires:
  bus_in/1 -> slice4/1, slice3/1, slice2/1, slice1/1
  slice4/1 -> reinterpret4/1
  reinterpret4/1 -> msb_out4/1
  slice3/1 -> reinterpret3/1
  reinterpret3/1 -> out3/1
  slice2/1 -> reinterpret2/1
  reinterpret2/1 -> out2/1
  slice1/1 -> reinterpret1/1
  reinterpret1/1 -> lsb_out1/1

== system pfb_fft_vacc_float/ss_pfb_sq0/ss/add_gen/edge_detect ==
  in : Inport
  Delay : xbsIndex_r4/Delay  rst=off  infoeditControl=Selection of Reset will increase slice count due to use of real FFs and instead of SRLs  en=off  latency=1  reg_retiming=off  dbl_ovrd=off  block_type=delay
      settings: xl_area=[0, 0, 0, 0, 0, 0, 0]
  Inverter : xbsIndex_r4/Inverter  en=off  latency=0  dbl_ovrd=off  block_type=inv
      settings: xl_area=[0, 0, 0, 0, 0, 0, 0]
  edge_op : xbsIndex_r4/Logical  logical_function=XNOR  inputs=2  en=off  latency=0  precision=Full  arith_type=Unsigned  n_bits=8  bin_pt=2  align_bp=on  dbl_ovrd=off  block_type=logical
      settings: xl_area=[0, 0, 0, 0, 0, 0, 0]
  out : Outport
  wires:
  Delay/1 -> edge_op/2
  in/1 -> Delay/1, Inverter/1
  edge_op/1 -> out/1
  Inverter/1 -> edge_op/1

== system pfb_fft_vacc_float/ss_pfb_sq0/ss/add_gen/edge_detect1 ==
  in : Inport
  Delay : xbsIndex_r4/Delay  rst=off  infoeditControl=Selection of Reset will increase slice count due to use of real FFs and instead of SRLs  en=off  latency=1  reg_retiming=off  dbl_ovrd=off  block_type=delay
      settings: xl_area=[0, 0, 0, 0, 0, 0, 0]
  Inverter : xbsIndex_r4/Inverter  en=off  latency=0  dbl_ovrd=off  block_type=inv
      settings: xl_area=[0, 0, 0, 0, 0, 0, 0]
  edge_op : xbsIndex_r4/Logical  logical_function=XNOR  inputs=2  en=off  latency=0  precision=Full  arith_type=Unsigned  n_bits=8  bin_pt=2  align_bp=on  dbl_ovrd=off  block_type=logical
      settings: xl_area=[0, 0, 0, 0, 0, 0, 0]
  out : Outport
  wires:
  Inverter/1 -> edge_op/1
  edge_op/1 -> out/1
  in/1 -> Inverter/1, Delay/1
  Delay/1 -> edge_op/2

== system pfb_fft_vacc_float/ss_pfb_sq0/ss/basic_ctrl/dram_munge ==
  note: 1st output same time
      | as last input
  note: Choose DRAM data
      | or standard. Constant input
      | to muxes should ensure
      | other path optimised
      | away
  note: Buffer 4 input words when using DRAM
      | then combine and output the first two,
      | then second. Ensures that a full burst
      | goes through every time so that status
      | register records number of bytes fully in
      | DRAM
  vin : Inport
  din : Inport  Port=2
  we : Inport  Port=3
  init : Inport  Port=4
  Concat : xbsIndex_r4/Concat  num_inputs=4  dbl_ovrd=off  block_type=concat
  Concat1 : xbsIndex_r4/Concat  num_inputs=4  dbl_ovrd=off  block_type=concat
  Constant : xbsIndex_r4/Constant  const=0  gui_display_data_type=Fixed-point  arith_type=Unsigned  n_bits=8  bin_pt=0  preci_type=Single  exp_width=8  frac_width=24  explicit_period=on  period=1  equ=P=C  opselect=C  inp2=PCIN>>17  opr=+  inp1=P  carry=CIN  dbl_ovrd=off  block_type=constant
      settings: block_version=11.4
  Delay : xbsIndex_r4/Delay  rst=off  infoeditControl=Selection of Reset will increase slice count due to use of real FFs and instead of SRLs  en=off  latency=1  reg_retiming=off  dbl_ovrd=off  block_type=delay
      settings: block_version=11.4
  Delay1 : xbsIndex_r4/Delay  rst=off  infoeditControl=Selection of Reset will increase slice count due to use of real FFs and instead of SRLs  en=off  latency=1  reg_retiming=off  dbl_ovrd=off  block_type=delay
      settings: block_version=11.4
  Logical : xbsIndex_r4/Logical  logical_function=AND  inputs=2  en=off  latency=1  precision=Full  arith_type=Unsigned  n_bits=16  bin_pt=0  align_bp=on  dbl_ovrd=off  block_type=logical
      settings: block_version=11.4
  Logical1 : xbsIndex_r4/Logical  logical_function=OR  inputs=2  en=off  latency=0  precision=Full  arith_type=Unsigned  n_bits=16  bin_pt=0  align_bp=on  dbl_ovrd=off  block_type=logical
      settings: block_version=11.4
  Logical2 : xbsIndex_r4/Logical  logical_function=AND  inputs=2  en=off  latency=0  precision=Full  arith_type=Unsigned  n_bits=16  bin_pt=0  align_bp=on  dbl_ovrd=off  block_type=logical
      settings: block_version=11.4
  Mux1 : xbsIndex_r4/Mux  inputs=2  en=off  latency=1  precision=Full  arith_type=Unsigned  n_bits=16  bin_pt=14  quantization=Truncate  overflow=Wrap  dbl_ovrd=off  block_type=mux
      settings: block_version=11.4
  Register{,1..3,5} (5 alike) : xbsIndex_r4/Register  init=0  rst=off  en=on  dbl_ovrd=off  block_type=register
      settings: block_version=11.4
  Register4 : xbsIndex_r4/Register  init=0  rst=on  en=on  dbl_ovrd=off  block_type=register
      settings: block_version=11.4
  Relational{,1..3} (4 alike) : xbsIndex_r4/Relational  mode=a=b  op_type=Bool  en=off  latency=0  dbl_ovrd=off  block_type=relational
  con0 : xbsIndex_r4/Constant  const=0  gui_display_data_type=Fixed-point  arith_type=Unsigned  n_bits=2  bin_pt=0  preci_type=Single  exp_width=8  frac_width=24  explicit_period=on  period=1  equ=P=C  opselect=C  inp2=PCIN>>17  opr=+  inp1=P  carry=CIN  dbl_ovrd=off  block_type=constant
      settings: block_version=11.4
  con1 : xbsIndex_r4/Constant  const=1  gui_display_data_type=Fixed-point  arith_type=Unsigned  n_bits=2  bin_pt=0  preci_type=Single  exp_width=8  frac_width=24  explicit_period=on  period=1  equ=P=C  opselect=C  inp2=PCIN>>17  opr=+  inp1=P  carry=CIN  dbl_ovrd=off  block_type=constant
      settings: block_version=11.4
  con2 : xbsIndex_r4/Constant  const=2  gui_display_data_type=Fixed-point  arith_type=Unsigned  n_bits=2  bin_pt=0  preci_type=Single  exp_width=8  frac_width=24  explicit_period=on  period=1  equ=P=C  opselect=C  inp2=PCIN>>17  opr=+  inp1=P  carry=CIN  dbl_ovrd=off  block_type=constant
      settings: block_version=11.4
  con3 : xbsIndex_r4/Constant  const=3  gui_display_data_type=Fixed-point  arith_type=Unsigned  n_bits=2  bin_pt=0  preci_type=Single  exp_width=8  frac_width=24  explicit_period=on  period=1  equ=P=C  opselect=C  inp2=PCIN>>17  opr=+  inp1=P  carry=CIN  dbl_ovrd=off  block_type=constant
      settings: block_version=11.4
  data_choice : xbsIndex_r4/Mux  inputs=2  en=off  latency=0  precision=User Defined  arith_type=Unsigned  n_bits=32  bin_pt=0  quantization=Truncate  overflow=Wrap  dbl_ovrd=off  block_type=mux
      settings: block_version=11.4
  dout_count : xbsIndex_r4/Counter  cnt_type=Free Running  cnt_to=Inf  operation=Up  start_count=0  cnt_by_val=1  arith_type=Unsigned  n_bits=1  bin_pt=0  load_pin=off  rst=on  en=on  explicit_period=on  period=1  use_behavioral_HDL=off  implementation=Fabric  dbl_ovrd=off  block_type=counter
      settings: block_version=11.4
  dram : xbsIndex_r4/Constant  const=0  gui_display_data_type=Fixed-point  arith_type=Boolean  n_bits=16  bin_pt=14  preci_type=Single  exp_width=8  frac_width=24  explicit_period=on  period=1  equ=P=C  opselect=C  inp2=PCIN>>17  opr=+  inp1=P  carry=CIN  dbl_ovrd=off  block_type=constant
      settings: block_version=11.4
  input_count : xbsIndex_r4/Counter  cnt_type=Free Running  cnt_to=Inf  operation=Up  start_count=0  cnt_by_val=1  arith_type=Unsigned  n_bits=2  bin_pt=0  load_pin=off  rst=on  en=on  explicit_period=on  period=1  use_behavioral_HDL=off  implementation=Fabric  dbl_ovrd=off  block_type=counter
      settings: block_version=11.4
  vout_choice : xbsIndex_r4/Mux  inputs=2  en=off  latency=0  precision=Full  arith_type=Unsigned  n_bits=16  bin_pt=14  quantization=Truncate  overflow=Wrap  dbl_ovrd=off  block_type=mux
      settings: block_version=11.4
  we_choice : xbsIndex_r4/Mux  inputs=2  en=off  latency=0  precision=Full  arith_type=Unsigned  n_bits=16  bin_pt=14  quantization=Truncate  overflow=Wrap  dbl_ovrd=off  block_type=mux
      settings: block_version=11.4
  vout : Outport
  dout : Outport  Port=2
  we_o : Outport  Port=3
  wires:
  Register3/1 -> Concat1/4
  Register2/1 -> Concat1/2
  Constant/1 -> Concat1/3, Concat1/1, Concat/3, Concat/1
  Register1/1 -> Concat/4
  Register/1 -> Concat/2
  [vin] -> vout_choice/2
  Register5/1 -> vout_choice/3
  vout_choice/1 -> vout/1
  Logical2/1 -> Register5/2
  [we] -> Logical2/2, Register4/2
  vin/1 -> [vin], Register5/1
  Register4/1 -> Logical2/1
  [init] -> Register4/3, Register4/1
  dram/1 -> vout_choice/1, we_choice/1, data_choice/1
  [din] -> data_choice/2
  Mux1/1 -> data_choice/3
  [we] -> we_choice/2
  Delay1/1 -> we_choice/3
  we_choice/1 -> we_o/1
  dout_count/1 -> Mux1/1
  [init] -> dout_count/1
  Logical1/1 -> Delay1/1, dout_count/2
  Concat1/1 -> Mux1/3
  Concat/1 -> Mux1/2
  Delay/1 -> Logical1/2
  Logical/1 -> Logical1/1, Delay/1
  Relational3/1 -> Logical/1, Register3/2
  Relational2/1 -> Register2/2
  Relational1/1 -> Register1/2
  Relational/1 -> Register/2
  con3/1 -> Relational3/2
  con2/1 -> Relational2/2
  con1/1 -> Relational1/2
  con0/1 -> Relational/2
  input_count/1 -> Relational3/1, Relational2/1, Relational1/1, Relational/1
  din/1 -> [din], Register3/1, Register2/1, Register1/1, Register/1
  we/1 -> [we], Logical/2, input_count/2
  init/1 -> [init], input_count/1
  data_choice/1 -> dout/1
